simulink library "lib_all"  (Simulink 7.6)
settings: Solver=ode45  SolverMode=SingleTasking  StartTime=0.0  StopTime=10.0  RelTol=1e-3
values from neighbouring files (symbols this model uses):
  map_lib_03 = [6.8679 7.0159 7.4571 8.1849 9.1892 10.456 11.97 13.71 15.655 17.781 20.063 22.474 ... (803 elements, 73x11)]    [scr_simdata.mat]    (also in scr_simdata_all.mat)
  map_lib_06 = [2.5784 2.6727 2.9588 3.4453 4.1444 5.068 6.226 7.6242 9.2634 11.139 13.24 15.552 ... (803 elements, 73x11)]    [scr_simdata.mat]    (also in scr_simdata_all.mat)
  map_lib_09 = [0.0025784 0.0026727 0.0029588 0.0034453 0.0041444 0.005068 0.006226 0.0076242 0.0092634 0.011139 0.01324 0.015552 ... (803 elements, 73x11)]    [scr_simdata.mat]    (also in scr_simdata_all.mat)
  map_lib_10 = [2.74 2.75 2.76 2.77 2.78 2.79 2.8 2.81 2.82 2.83 2.84 2.85 ... (81 elements, 1x81)]    [scr_simdata.mat]    (also in scr_simdata_all.mat)
  map_lib_11 = [1.1983 1.2027 1.207 1.2113 1.2156 1.2198 1.2241 1.2283 1.2326 1.2368 1.241 1.2451 ... (81 elements, 1x81)]    [scr_simdata.mat]    (also in scr_simdata_all.mat)
  map_lib_12 = [2.74 2.75 2.76 2.77 2.78 2.79 2.8 2.81 2.82 2.83 2.84 2.85 ... (81 elements, 1x81)]    [scr_simdata.mat]    (also in scr_simdata_all.mat)
  map_lib_13 = [1.74 1.75 1.76 1.77 1.78 1.79 1.8 1.81 1.82 1.83 1.84 1.85 ... (81 elements, 1x81)]    [scr_simdata.mat]    (also in scr_simdata_all.mat)
  map_lib_16 = [0.00048828125 1.6159 1.8962 2.0821 2.2251 2.3426 2.4433 2.5318 2.611 2.683 2.749 2.8101 ... (1419 elements, 129x11)]    [scr_simdata.mat]    (also in scr_simdata_all.mat)
  map_mr2_01 = [0 800 1600 2400 3200 4000]    [scr_simdata.mat]    (also in scr_simdata_all.mat)
  map_mr2_02 = [1.32 1.32 1.33 1.34 1.36 1.38]    [scr_simdata.mat]    (also in scr_simdata_all.mat)
  map_mr2_03 = [0 800 1600 2400 3200 4000]    [scr_simdata.mat]    (also in scr_simdata_all.mat)
  map_mr2_04 = [1.32 1.32 1.33 1.34 1.36 1.38]    [scr_simdata.mat]    (also in scr_simdata_all.mat)
  map_mr2_05 = [0 800 1600 2400 3200 4000]    [scr_simdata.mat]    (also in scr_simdata_all.mat)
  map_mr2_06 = [1.32 1.32 1.33 1.34 1.36 1.38]    [scr_simdata.mat]    (also in scr_simdata_all.mat)
  Parameter1 = 6    [scr_simdata.mat]    (also in scr_simdata_all.mat)
  Parameter101 = 30    [scr_simdata.mat]    (also in scr_simdata_all.mat)
  Parameter102 = 12    [scr_simdata.mat]    (also in scr_simdata_all.mat)
  Parameter103 = -240    [scr_simdata.mat]    (also in scr_simdata_all.mat)
  Parameter108 = 48    [scr_simdata.mat]    (also in scr_simdata_all.mat)
  Parameter120 = 16    [scr_simdata.mat]    (also in scr_simdata_all.mat)
  Parameter121 = 87    [scr_simdata.mat]    (also in scr_simdata_all.mat)
  Parameter123 = 78    [scr_simdata.mat]    (also in scr_simdata_all.mat)
  Parameter124 = 60    [scr_simdata.mat]    (also in scr_simdata_all.mat)
  Parameter125 = -480    [scr_simdata.mat]    (also in scr_simdata_all.mat)
  Parameter130 = 96    [scr_simdata.mat]    (also in scr_simdata_all.mat)
  Parameter131 = 3    [scr_simdata.mat]    (also in scr_simdata_all.mat)
  Parameter144 = 3    [scr_simdata.mat]    (also in scr_simdata_all.mat)
  Parameter18 = 128    [scr_simdata.mat]    (also in scr_simdata_all.mat)
  Parameter19 = 16    [scr_simdata.mat]    (also in scr_simdata_all.mat)
  Parameter2 = 12    [scr_simdata.mat]    (also in scr_simdata_all.mat)
  Parameter20 = 7    [scr_simdata.mat]    (also in scr_simdata_all.mat)
  Parameter21 = 4    [scr_simdata.mat]    (also in scr_simdata_all.mat)
  Parameter3 = 18    [scr_simdata.mat]    (also in scr_simdata_all.mat)
  Parameter32 = 1    [scr_simdata.mat]    (also in scr_simdata_all.mat)
  Parameter34 = 24    [scr_simdata.mat]    (also in scr_simdata_all.mat)
  Parameter4 = 138    [scr_simdata.mat]    (also in scr_simdata_all.mat)
  Parameter42 = 48    [scr_simdata.mat]    (also in scr_simdata_all.mat)
  Parameter5 = 135    [scr_simdata.mat]    (also in scr_simdata_all.mat)
  Parameter55 = 0    [scr_simdata.mat]    (also in scr_simdata_all.mat)
  Parameter56 = 0    [scr_simdata.mat]    (also in scr_simdata_all.mat)
  Parameter57 = 1    [scr_simdata.mat]    (also in scr_simdata_all.mat)
  Parameter58 = 1    [scr_simdata.mat]    (also in scr_simdata_all.mat)
  Parameter59 = 2    [scr_simdata.mat]    (also in scr_simdata_all.mat)
  Parameter6 = 132    [scr_simdata.mat]    (also in scr_simdata_all.mat)
  Parameter60 = 2    [scr_simdata.mat]    (also in scr_simdata_all.mat)
  Parameter63 = 144    [scr_simdata.mat]    (also in scr_simdata_all.mat)
  Parameter7 = 126    [scr_simdata.mat]    (also in scr_simdata_all.mat)
  Parameter76 = 64    [scr_simdata.mat]    (also in scr_simdata_all.mat)
  Parameter77 = 135    [scr_simdata.mat]    (also in scr_simdata_all.mat)
  Parameter79 = 126    [scr_simdata.mat]    (also in scr_simdata_all.mat)
  Parameter80 = 108    [scr_simdata.mat]    (also in scr_simdata_all.mat)
  Parameter81 = 0    [scr_simdata.mat]    (also in scr_simdata_all.mat)
  Parameter86 = 0    [scr_simdata.mat]    (also in scr_simdata_all.mat)
  Parameter98 = 112    [scr_simdata.mat]    (also in scr_simdata_all.mat)
  Parameter99 = 39    [scr_simdata.mat]    (also in scr_simdata_all.mat)
undefined symbols (not defined in the file or in its folder): cylvolkp_hih_map2h, cylvolkp_map2h, pk1k_map2h
libraries: simulink x2
(part 1 of 36)
settings Model: autoc_cdm_savetype=ParentModel  autoc_cdm_name=database_dummy  autoc_current_model=lib_all  Creator=112454  LastModifiedBy=fukudak

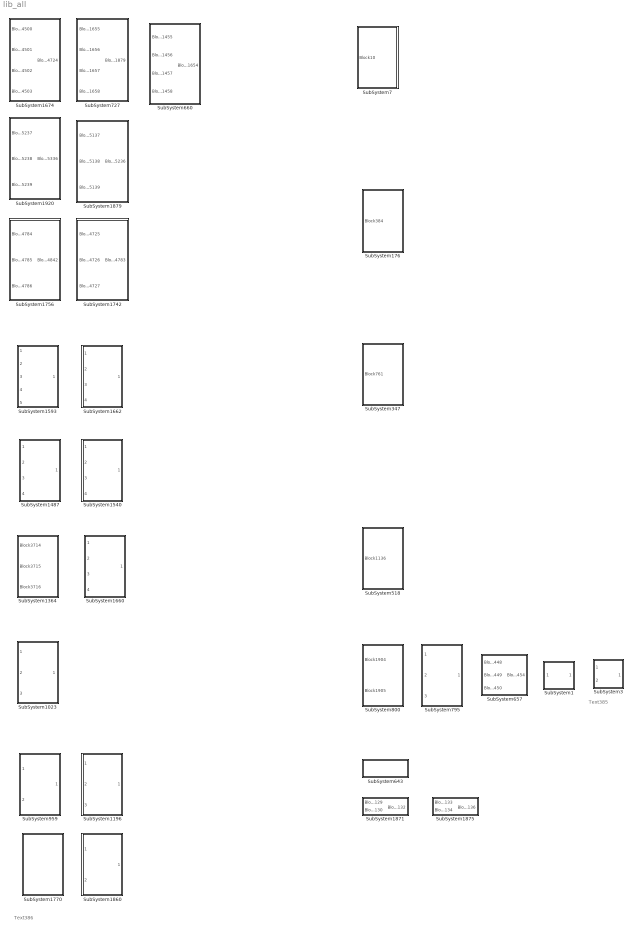
== system lib_all ==
  note: Text386
  note: Text385
  SubSystem1 : Subsystem  TreatAsAtomicUnit=on
  SubSystem1023 : Subsystem  TreatAsAtomicUnit=on
      settings: AttributesFormatString=Attribute523  RTWFcnNameOpts=Use subsystem name
  SubSystem1196 : Subsystem  TreatAsAtomicUnit=on
      settings: AttributesFormatString=Attribute636  RTWFcnNameOpts=Use subsystem name
  SubSystem1364 : Subsystem  TreatAsAtomicUnit=on
      settings: AttributesFormatString=Attribute739  RTWFcnNameOpts=Use subsystem name
  SubSystem1487 : Subsystem  TreatAsAtomicUnit=on
      settings: AttributesFormatString=Attribute793  RTWFcnNameOpts=Use subsystem name
  SubSystem1540 : Subsystem  TreatAsAtomicUnit=on
      settings: AttributesFormatString=Attribute812  RTWFcnNameOpts=Use subsystem name
  SubSystem1593 : Subsystem  TreatAsAtomicUnit=on
      settings: AttributesFormatString=Attribute831  RTWFcnNameOpts=Use subsystem name
  SubSystem1660 : Subsystem  TreatAsAtomicUnit=on
      settings: AttributesFormatString=Attribute864  RTWFcnNameOpts=Use subsystem name
  SubSystem1662 : Subsystem  TreatAsAtomicUnit=on
      settings: AttributesFormatString=Attribute867  RTWFcnNameOpts=Use subsystem name
  SubSystem1674 : Subsystem  TreatAsAtomicUnit=on
      settings: AttributesFormatString=Attribute880  RTWFcnNameOpts=Use subsystem name
  SubSystem1742 : Subsystem  TreatAsAtomicUnit=on
      settings: AttributesFormatString=Attribute917  RTWFcnNameOpts=Use subsystem name
  SubSystem1756 : Subsystem  TreatAsAtomicUnit=on
      settings: AttributesFormatString=Attribute931  RTWFcnNameOpts=Use subsystem name
  SubSystem176 : Subsystem  TreatAsAtomicUnit=on
      settings: AttributesFormatString=Attribute82  RTWFcnNameOpts=Use subsystem name
  SubSystem1770 : Subsystem  TreatAsAtomicUnit=on
      settings: AttributesFormatString=Attribute945  RTWFcnNameOpts=Use subsystem name
  SubSystem1860 : Subsystem  TreatAsAtomicUnit=on
      settings: AttributesFormatString=Attribute1028  RTWFcnNameOpts=Use subsystem name
  SubSystem1871 : Subsystem
  SubSystem1875 : Subsystem
  SubSystem1879 : Subsystem  TreatAsAtomicUnit=on
      settings: AttributesFormatString=Attribute1038  RTWFcnNameOpts=Use subsystem name
  SubSystem1920 : Subsystem  TreatAsAtomicUnit=on
      settings: AttributesFormatString=Attribute1054  RTWFcnNameOpts=Use subsystem name
  SubSystem3 : Subsystem
  SubSystem347 : Subsystem  TreatAsAtomicUnit=on
      settings: AttributesFormatString=Attribute164  RTWFcnNameOpts=Use subsystem name
  SubSystem518 : Subsystem  TreatAsAtomicUnit=on
      settings: AttributesFormatString=Attribute245  RTWFcnNameOpts=Use subsystem name
  SubSystem643 : Subsystem  TreatAsAtomicUnit=on  RTWFileName=gacrnk_get
      settings: RTWFcnNameOpts=Use subsystem name
  SubSystem657 : Subsystem  TreatAsAtomicUnit=on
      settings: RTWSystemCode=Inline  RTWFcnNameOpts=Use subsystem name
  SubSystem660 : Subsystem  TreatAsAtomicUnit=on
      settings: AttributesFormatString=Attribute306  RTWFcnNameOpts=Use subsystem name
  SubSystem7 : Subsystem  TreatAsAtomicUnit=on
      settings: AttributesFormatString=Attribute2  RTWFcnNameOpts=Use subsystem name
  SubSystem727 : Subsystem  TreatAsAtomicUnit=on
      settings: AttributesFormatString=Attribute342  RTWFcnNameOpts=Use subsystem name
  SubSystem795 : Subsystem  TreatAsAtomicUnit=on
      settings: AttributesFormatString=Attribute379  RTWFcnNameOpts=Use subsystem name
  SubSystem800 : Subsystem  TreatAsAtomicUnit=on
      settings: AttributesFormatString=Attribute386  RTWFcnNameOpts=Use subsystem name
  SubSystem959 : Subsystem  TreatAsAtomicUnit=on
      settings: AttributesFormatString=Attribute491  RTWFcnNameOpts=Use subsystem name

== system lib_all/SubSystem1 ==
  note: Text1
  Block1 : Inport
  Block2 : Constant  Value=Parameter63  OutDataTypeStr=double  SampleTime=-1
  Block3 : Sum  Inputs=+-  OutDataTypeStr=double  RndMeth=Zero  SaturateOnIntegerOverflow=on  InputSameDT=on
  Block4 : Switch  Threshold=0.5  RndMeth=Zero  SaturateOnIntegerOverflow=on  InputSameDT=on
  SubSystem2 : Subsystem
  Block5 : Outport
  wires:
  Block2/1 -> Block3/2, SubSystem2/2(In2)
  SubSystem2/1(Out1) -> Block4/2
  Block3/1 -> Block4/1
  Block1/1 -> SubSystem2/1(In1), Block3/1, Block4/3
  Block4/1 -> Block5/1

== system lib_all/SubSystem1023 ==
  note: Text236
  Block2623 : Inport
  Block2624 : Inport  Port=2
  Block2625 : Inport  Port=3
  Block2626 : Constant  Value=0  OutDataTypeStr=double  SampleTime=-1
  Block2627 : Constant  Value=0  OutDataTypeStr=double  SampleTime=-1
  Block2628 : Sum  Inputs=-+  OutDataTypeStr=double  RndMeth=Zero  SaturateOnIntegerOverflow=on  InputSameDT=on
  Block2629 : Sum  Inputs=+-  OutDataTypeStr=double  RndMeth=Zero  SaturateOnIntegerOverflow=on  InputSameDT=on
  SubSystem1024 : Subsystem  TreatAsAtomicUnit=on  mask(***ATC.DIVISION***){bd_version=1.01.A, r0=チェック無し, r1=, r2=, r3=, r4=, r5=, r6=, r7=, r8=, r9=, c0=, c1=, c2=, c3=, c4=, c5=, c6=, c7=, c8=, c9=}
      mask prompts: bd_version "Version"
      mask help: これはAutoCからのブロックです．編集禁止．
      settings: OpenFcn=warndlg('変更禁止!');
      mask Help: web(ui_blockhelpmng(gcb));
  SubSystem1025 : Subsystem  Priority=1  TreatAsAtomicUnit=on
      settings: AttributesFormatString=Attribute526  RTWFcnNameOpts=Use subsystem name
  SubSystem1093 : Subsystem  Priority=2  TreatAsAtomicUnit=on
      settings: AttributesFormatString=Attribute563  RTWFcnNameOpts=Use subsystem name
  SubSystem1161 : Subsystem  Priority=3  TreatAsAtomicUnit=on
      settings: AttributesFormatString=Attribute600  RTWFcnNameOpts=Use subsystem name
  SubSystem1175 : Subsystem  Priority=4  TreatAsAtomicUnit=on
      settings: AttributesFormatString=Attribute614  RTWFcnNameOpts=Use subsystem name
  SubSystem1189 : Subsystem
  Block3216 : Outport
  wires:
  Block2626/1 -> SubSystem1025/4(Block2633)
  Block2627/1 -> SubSystem1093/4(Block2858)
  SubSystem1189/1(Block3215) -> SubSystem1025/3(Block2632), SubSystem1093/3(Block2857), SubSystem1175/3(Block3141), SubSystem1161/3(Block3082)
  Block2625/1 -> SubSystem1175/2(Block3140), SubSystem1093/2(Block2856)
  Block2624/1 -> SubSystem1161/2(Block3081), SubSystem1025/2(Block2631)
  SubSystem1024/1(Out) -> Block3216/1
  Block2629/1 -> SubSystem1024/2(div)
  Block2628/1 -> SubSystem1024/1(mult)
  SubSystem1175/1(Block3197) -> Block2629/2
  SubSystem1161/1(Block3138) -> Block2629/1
  SubSystem1093/1(Block3079) -> Block2628/2
  SubSystem1025/1(Block2854) -> Block2628/1
  Block2623/1 -> SubSystem1025/1(Block2630), SubSystem1093/1(Block2855), SubSystem1175/1(Block3139), SubSystem1161/1(Block3080), SubSystem1189/1(Block3198)

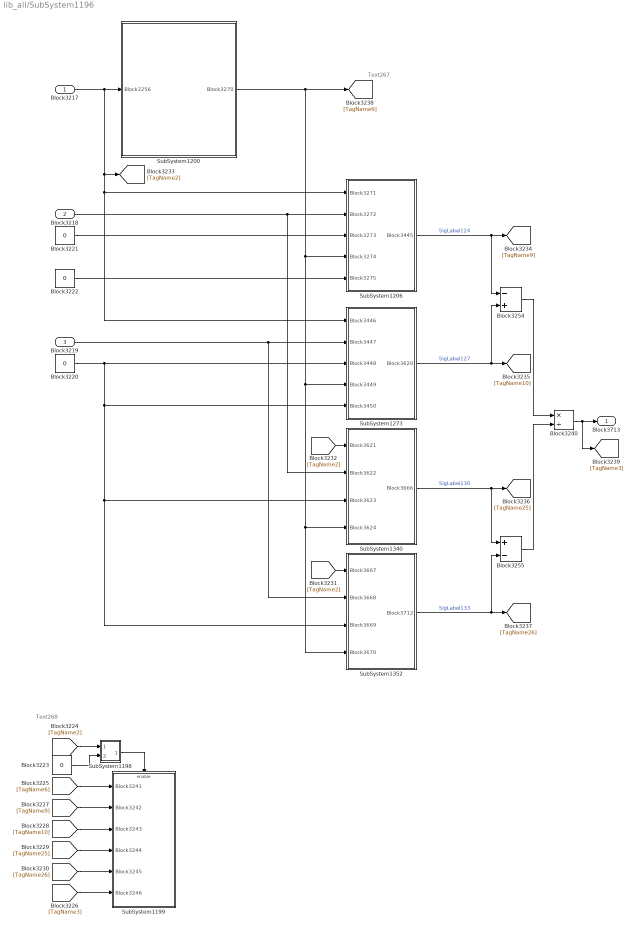
== system lib_all/SubSystem1196 ==
  note: Text268
  note: Text267
  Block3217 : Inport
  Block3218 : Inport  Port=2
  Block3219 : Inport  Port=3
  Block{3220..3223} (4 alike) : Constant  Value=0  OutDataTypeStr=double  SampleTime=-1
  Block3240 : Product  Inputs=*/  OutDataTypeStr=double  SaturateOnIntegerOverflow=on  RndMeth=Zero  InputSameDT=on
  Block3254 : Sum  Inputs=-+  OutDataTypeStr=double  RndMeth=Zero  SaturateOnIntegerOverflow=on  InputSameDT=on
  Block3255 : Sum  Inputs=+-  OutDataTypeStr=double  RndMeth=Zero  SaturateOnIntegerOverflow=on  InputSameDT=on
  SubSystem1198 : Subsystem
  SubSystem1199 : Subsystem
  SubSystem1200 : Subsystem
  SubSystem1206 : Subsystem  Priority=1  TreatAsAtomicUnit=on
      settings: AttributesFormatString=Attribute647  RTWFcnNameOpts=Use subsystem name
  SubSystem1273 : Subsystem  Priority=2  TreatAsAtomicUnit=on
      settings: AttributesFormatString=Attribute680  RTWFcnNameOpts=Use subsystem name
  SubSystem1340 : Subsystem  Priority=3  TreatAsAtomicUnit=on
      settings: AttributesFormatString=Attribute713  RTWFcnNameOpts=Use subsystem name
  SubSystem1352 : Subsystem  Priority=4  TreatAsAtomicUnit=on
      settings: AttributesFormatString=Attribute726  RTWFcnNameOpts=Use subsystem name
  Block3713 : Outport
  wires:
  [TagName2] -> SubSystem1340/1(Block3621)
  [TagName2] -> SubSystem1352/1(Block3667)
  SubSystem1198/1(Out1) -> SubSystem1199/Enable
  [TagName26] -> SubSystem1199/5(Block3245)
  [TagName25] -> SubSystem1199/4(Block3244)
  [TagName10] -> SubSystem1199/3(Block3243)
  [TagName9] -> SubSystem1199/2(Block3242)
  [TagName3] -> SubSystem1199/6(Block3246)
  [TagName6] -> SubSystem1199/1(Block3241)
  Block3223/1 -> SubSystem1198/2(In2)
  [TagName2] -> SubSystem1198/1(In1)
  Block3240/1 -> Block3713/1, [TagName3]
  Block3255/1 -> Block3240/2
  Block3254/1 -> Block3240/1
  SubSystem1352/1(Block3712) -> Block3255/2, [TagName26]  "SigLabel133"
  SubSystem1340/1(Block3666) -> Block3255/1, [TagName25]  "SigLabel130"
  SubSystem1273/1(Block3620) -> Block3254/2, [TagName10]  "SigLabel127"
  SubSystem1206/1(Block3445) -> Block3254/1, [TagName9]  "SigLabel124"
  Block3220/1 -> SubSystem1273/3(Block3448), SubSystem1273/5(Block3450), SubSystem1352/3(Block3669), SubSystem1340/3(Block3623)
  Block3219/1 -> SubSystem1352/2(Block3668), SubSystem1273/2(Block3447)
  SubSystem1200/1(Block3270) -> SubSystem1206/4(Block3274), SubSystem1352/4(Block3670), SubSystem1340/4(Block3624), SubSystem1273/4(Block3449), [TagName6]
  Block3222/1 -> SubSystem1206/5(Block3275)
  Block3221/1 -> SubSystem1206/3(Block3273)
  Block3218/1 -> SubSystem1340/2(Block3622), SubSystem1206/2(Block3272)
  Block3217/1 -> SubSystem1200/1(Block3256), SubSystem1273/1(Block3446), SubSystem1206/1(Block3271), [TagName2]

== system lib_all/SubSystem1364 ==
  note: Text289
  note: Text288
  note: Text287
  note: Text286
  Block3714 : Inport
  Block3715 : Inport  Port=2
  Block3716 : Inport  Port=3
  Block3717 : Constant  Value=0  OutDataTypeStr=double  SampleTime=-1
  Block3718 : Constant  OutDataTypeStr=double  SampleTime=-1
  Block3719 : Constant  Value=0  OutDataTypeStr=double  SampleTime=-1
  Block3720 : DataStoreRead  DataStoreName=DSM15
  Block3721 : DataStoreWrite  DataStoreName=DSM101
  Block3730 : Sum  OutDataTypeStr=double  RndMeth=Zero  SaturateOnIntegerOverflow=on  InputSameDT=on
  SubSystem1366 : Subsystem  Priority=1  TreatAsAtomicUnit=on
      settings: AttributesFormatString=Attribute743  RTWFcnNameOpts=Use subsystem name
  SubSystem1419 : Subsystem  Priority=2  TreatAsAtomicUnit=on
      settings: AttributesFormatString=Attribute762  RTWFcnNameOpts=Use subsystem name
  SubSystem1472 : Subsystem
  SubSystem1478 : Subsystem  TreatAsAtomicUnit=on
      settings: RTWFcnNameOpts=Use subsystem name
  SubSystem1480 : Subsystem  TreatAsAtomicUnit=on  mask(***ATC.DSWVEC***){bd_version=1.01.A, r0=DSM99, r1=-1, r2=, r3=, r4=, r5=, r6=, r7=, r8=, r9=, c0=, c1=, c2=, c3=, c4=, c5=, c6=, c7=, c8=, c9=}
      mask prompts: bd_version "Version"
      mask help: これはAutoCからのブロックです．編集禁止．
      settings: OpenFcn=warndlg('変更禁止!');
      mask Help: web(ui_blockhelpmng(gcb));
      mask Display: disp('ATC.DSRVEC')
  SubSystem1481 : Subsystem  TreatAsAtomicUnit=on  mask(***ATC.DSWVEC***){bd_version=1.01.A, r0=DSM100, r1=-1, r2=, r3=, r4=, r5=, r6=, r7=, r8=, r9=, c0=, c1=, c2=, c3=, c4=, c5=, c6=, c7=, c8=, c9=}
      mask prompts: bd_version "Version"
      mask help: これはAutoCからのブロックです．編集禁止．
      settings: OpenFcn=warndlg('変更禁止!');
      mask Help: web(ui_blockhelpmng(gcb));
      mask Display: disp('ATC.DSRVEC')
  SubSystem1482 : Subsystem  TreatAsAtomicUnit=on  mask(***ATC.DSRVEC***){bd_version=1.01.A, r0=DSM98, r1=-1, r2=, r3=, r4=, r5=, r6=, r7=, r8=, r9=, c0=, c1=, c2=, c3=, c4=, c5=, c6=, c7=, c8=, c9=}
      mask prompts: bd_version "Version"
      mask help: これはAutoCからのブロックです．編集禁止．
      settings: OpenFcn=warndlg('変更禁止!');
      mask Help: web(ui_blockhelpmng(gcb));
      mask Display: disp('ATC.DSRVEC')
  SubSystem1483 : Subsystem  TreatAsAtomicUnit=on  mask(***ATC.DSWVEC***){bd_version=1.01.A, r0=DSM41, r1=-1, r2=, r3=, r4=, r5=, r6=, r7=, r8=, r9=, c0=, c1=, c2=, c3=, c4=, c5=, c6=, c7=, c8=, c9=}
      mask prompts: bd_version "Version"
      mask help: これはAutoCからのブロックです．編集禁止．
      settings: OpenFcn=warndlg('変更禁止!');
      mask Help: web(ui_blockhelpmng(gcb));
      mask Display: disp('ATC.DSRVEC')
  SubSystem1484 : Subsystem  TreatAsAtomicUnit=on  mask(***ATC.DSRVEC***){bd_version=1.01.A, r0=DSM42, r1=-1, r2=, r3=, r4=, r5=, r6=, r7=, r8=, r9=, c0=, c1=, c2=, c3=, c4=, c5=, c6=, c7=, c8=, c9=}
      mask prompts: bd_version "Version"
      mask help: これはAutoCからのブロックです．編集禁止．
      settings: OpenFcn=warndlg('変更禁止!');
      mask Help: web(ui_blockhelpmng(gcb));
      mask Display: disp('ATC.DSRVEC')
  SubSystem1485 : Subsystem  TreatAsAtomicUnit=on  mask(***ATC.DSWVEC***){bd_version=1.01.A, r0=DSM42, r1=-1, r2=, r3=, r4=, r5=, r6=, r7=, r8=, r9=, c0=, c1=, c2=, c3=, c4=, c5=, c6=, c7=, c8=, c9=}
      mask prompts: bd_version "Version"
      mask help: これはAutoCからのブロックです．編集禁止．
      settings: OpenFcn=warndlg('変更禁止!');
      mask Help: web(ui_blockhelpmng(gcb));
      mask Display: disp('ATC.DSRVEC')
  SubSystem1486 : Subsystem  TreatAsAtomicUnit=on
  wires:
  [TagName17] -> SubSystem1480/1(input)
  [TagName16] -> SubSystem1481/1(input)
  [TagName5] -> SubSystem1480/2(element), SubSystem1481/2(element)
  [TagName15] -> Block3721/1
  Block3730/1 -> SubSystem1472/1(Block3975), SubSystem1485/2(element), SubSystem1483/2(element), SubSystem1482/1(element), SubSystem1484/1(element), [TagName5]
  SubSystem1472/1(Block3994) -> SubSystem1478/4(Block3999), [TagName15]
  SubSystem1486/1(Block4019) -> SubSystem1485/1(input)
  Block3720/1 -> SubSystem1486/3(Block4013)
  SubSystem1484/1(Output) -> SubSystem1486/1(Block4011)
  SubSystem1478/1(Block4010) -> SubSystem1486/2(Block4012), SubSystem1483/1(input)
  SubSystem1482/1(Output) -> SubSystem1478/3(Block3998)
  SubSystem1419/1(Block3974) -> SubSystem1478/2(Block3997), [TagName17]
  SubSystem1366/1(Block3852) -> SubSystem1478/1(Block3996), [TagName16]
  SubSystem1472/2(Block3995) -> SubSystem1419/4(Block3856), SubSystem1366/4(Block3734)
  Block3717/1 -> SubSystem1366/3(Block3733)
  Block3719/1 -> SubSystem1419/3(Block3855)
  Block3716/1 -> SubSystem1419/2(Block3854)
  Block3715/1 -> SubSystem1366/2(Block3732)
  Block3718/1 -> Block3730/2
  Block3714/1 -> Block3730/1, SubSystem1419/1(Block3853), SubSystem1366/1(Block3731)

== system lib_all/SubSystem1487 ==
  note: Text295
  Block4020 : Inport
  Block4021 : Inport  Port=2
  Block4022 : Inport  Port=3
  Block4023 : Inport  Port=4
  Block4024 : Constant  Value=0  OutDataTypeStr=double  SampleTime=-1
  Block4025 : Constant  OutDataTypeStr=double  SampleTime=-1
  Block4026 : Constant  Value=0  OutDataTypeStr=double  SampleTime=-1
  Block4027 : Gain  Gain=1/5  SaturateOnIntegerOverflow=on  OutDataTypeStr=Inherit: Same as input  ParamDataTypeStr=Inherit: Same as input
      settings: AttributesFormatString=Attribute797
  Block4028 : Product  OutDataTypeStr=double  SaturateOnIntegerOverflow=on  RndMeth=Zero  InputSameDT=on
  Block4029 : Sum  Inputs=-+  OutDataTypeStr=double  RndMeth=Zero  SaturateOnIntegerOverflow=on  InputSameDT=on
  Block4030 : Sum  OutDataTypeStr=double  RndMeth=Zero  SaturateOnIntegerOverflow=on  InputSameDT=on
  Block4031 : Sum  OutDataTypeStr=double  RndMeth=Zero  SaturateOnIntegerOverflow=on  InputSameDT=on
  Block4032 : Switch  Threshold=0.5  RndMeth=Zero  SaturateOnIntegerOverflow=on  InputSameDT=on
  SubSystem1489 : Subsystem
  SubSystem1490 : Subsystem
  SubSystem1496 : Subsystem
  Block4141 : Outport
  wires:
  Block4031/1 -> SubSystem1496/3(Block4062), SubSystem1490/2(Block4034)
  Block4020/1 -> Block4031/1
  Block4027/1 -> Block4030/2
  Block4028/1 -> Block4027/1
  Block4025/1 -> Block4031/2
  Block4024/1 -> Block4032/1
  Block4032/1 -> Block4141/1
  SubSystem1489/1(Out1) -> Block4032/2
  Block4026/1 -> SubSystem1489/2(In2)
  Block4022/1 -> SubSystem1490/3(Block4035)
  Block4030/1 -> SubSystem1489/1(In1), Block4032/3
  SubSystem1490/2(Block4059) -> Block4028/2
  Block4029/1 -> Block4028/1  "SigLabel142"
  SubSystem1496/2(Block4140) -> Block4029/2
  SubSystem1496/1(Block4139) -> Block4029/1, Block4030/1
  Block4023/1 -> SubSystem1496/2(Block4061)
  SubSystem1490/1(Block4058) -> SubSystem1496/1(Block4060)
  Block4021/1 -> SubSystem1490/1(Block4033)

== system lib_all/SubSystem1540 ==
  note: Text301
  Block4142 : Inport
  Block4143 : Inport  Port=2
  Block4144 : Inport  Port=3
  Block4145 : Inport  Port=4
  Block4146 : Constant  Value=0  OutDataTypeStr=double  SampleTime=-1
  Block4147 : Constant  OutDataTypeStr=double  SampleTime=-1
  Block4148 : Constant  Value=0  OutDataTypeStr=double  SampleTime=-1
  Block4149 : Gain  Gain=1/5  SaturateOnIntegerOverflow=on  OutDataTypeStr=Inherit: Same as input  ParamDataTypeStr=Inherit: Same as input
      settings: AttributesFormatString=Attribute816
  Block4150 : Product  OutDataTypeStr=double  SaturateOnIntegerOverflow=on  RndMeth=Zero  InputSameDT=on
  Block4151 : Sum  Inputs=-+  OutDataTypeStr=double  RndMeth=Zero  SaturateOnIntegerOverflow=on  InputSameDT=on
  Block4152 : Sum  OutDataTypeStr=double  RndMeth=Zero  SaturateOnIntegerOverflow=on  InputSameDT=on
  Block4153 : Sum  OutDataTypeStr=double  RndMeth=Zero  SaturateOnIntegerOverflow=on  InputSameDT=on
  Block4154 : Switch  Threshold=0.5  RndMeth=Zero  SaturateOnIntegerOverflow=on  InputSameDT=on
  SubSystem1542 : Subsystem
  SubSystem1543 : Subsystem
  SubSystem1549 : Subsystem
  Block4263 : Outport
  wires:
  Block4143/1 -> SubSystem1543/1(Block4155)
  SubSystem1543/1(Block4180) -> SubSystem1549/1(Block4182)
  Block4145/1 -> SubSystem1549/2(Block4183)
  SubSystem1549/1(Block4261) -> Block4152/1, Block4151/1
  SubSystem1549/2(Block4262) -> Block4151/2
  Block4151/1 -> Block4150/1  "SigLabel145"
  SubSystem1543/2(Block4181) -> Block4150/2
  Block4152/1 -> Block4154/3, SubSystem1542/1(In1)
  Block4144/1 -> SubSystem1543/3(Block4157)
  Block4148/1 -> SubSystem1542/2(In2)
  SubSystem1542/1(Out1) -> Block4154/2
  Block4154/1 -> Block4263/1
  Block4146/1 -> Block4154/1
  Block4147/1 -> Block4153/2
  Block4150/1 -> Block4149/1
  Block4149/1 -> Block4152/2
  Block4142/1 -> Block4153/1
  Block4153/1 -> SubSystem1543/2(Block4156), SubSystem1549/3(Block4184)

== system lib_all/SubSystem1593 ==
  note: Text313
  note: Text312
  note: Text311
  Block4264 : Inport
  Block4265 : Inport  Port=2
  Block4266 : Inport  Port=3
  Block4267 : Inport  Port=4
  Block4268 : Inport  Port=5
  Block4269 : Product  OutDataTypeStr=double  SaturateOnIntegerOverflow=on  RndMeth=Zero  InputSameDT=on
  SubSystem1595 : Subsystem  Priority=1  TreatAsAtomicUnit=on
      settings: AttributesFormatString=Attribute832  RTWFcnNameOpts=Use subsystem name
  SubSystem1648 : Subsystem  Priority=2  TreatAsAtomicUnit=on
      settings: AttributesFormatString=Attribute851  RTWFcnNameOpts=Use subsystem name
  Block4438 : Outport
  wires:
  Block4267/1 -> SubSystem1648/4(Block4395)
  Block4268/1 -> SubSystem1595/4(Block4273)
  Block4266/1 -> SubSystem1595/3(Block4272), SubSystem1648/3(Block4394)
  Block4269/1 -> Block4438/1
  SubSystem1648/1(Block4437) -> Block4269/2  "SigLabel151"
  SubSystem1595/1(Block4391) -> Block4269/1  "SigLabel150"
  Block4265/1 -> SubSystem1595/2(Block4271), SubSystem1648/2(Block4393)
  Block4264/1 -> SubSystem1595/1(Block4270), SubSystem1648/1(Block4392)

== system lib_all/SubSystem1660 ==
  note: Text316
  note: Text315
  note: Text314
  Block4439 : Inport
  Block4440 : Inport  Port=2
  Block4441 : Inport  Port=3
  Block4442 : Inport  Port=4
  Block4443 : DataStoreWrite  DataStoreName=DSM90
  Block4444 : DataStoreWrite  DataStoreName=DSM91
  Block4449 : Product  OutDataTypeStr=double  SaturateOnIntegerOverflow=on  RndMeth=Zero  InputSameDT=on
  Block4450 : Product  Inputs=*/  OutDataTypeStr=double  SaturateOnIntegerOverflow=on  RndMeth=Zero  InputSameDT=on
  Block4451 : Lookup2D  RowIndex=pk1k_map2h_y  ColumnIndex=pk1k_map2h_x  Table=pk1k_map2h  SaturateOnIntegerOverflow=on  InputSameDT=on
      settings: AttributesFormatString=<%<OutputValues>>\nAttribute865
  Block4452 : Lookup2D  RowIndex=pk1k_map2h_y  ColumnIndex=pk1k_map2h_x  Table=pk1k_map2h  SaturateOnIntegerOverflow=on  InputSameDT=on
      settings: AttributesFormatString=<%<OutputValues>>\nAttribute866
  Block4453 : Outport
  wires:
  Block4442/1 -> Block4451/2, Block4452/2
  [TagName20] -> Block4444/1
  [TagName19] -> Block4443/1
  Block4451/1 -> Block4450/2, [TagName19]
  Block4450/1 -> Block4453/1
  Block4449/1 -> Block4450/1
  Block4441/1 -> Block4449/2
  Block4452/1 -> Block4449/1, [TagName20]
  Block4439/1 -> Block4451/1
  Block4440/1 -> Block4452/1

== system lib_all/SubSystem1662 ==
  note: Text319
  Block4454 : Inport
  Block4455 : Inport  Port=2
  Block4456 : Inport  Port=3
  Block4457 : Inport  Port=4
  SubSystem1664 : Subsystem
  SubSystem1672 : Subsystem
  Block4499 : Outport
  wires:
  Block4456/1 -> SubSystem1664/3(Block4460)
  SubSystem1672/1(Block4498) -> Block4499/1
  Block4457/1 -> SubSystem1672/1(Block4491)
  SubSystem1664/1(Block4490) -> SubSystem1672/2(Block4492)
  Block4454/1 -> SubSystem1664/2(Block4459)
  Block4455/1 -> SubSystem1664/1(Block4458)

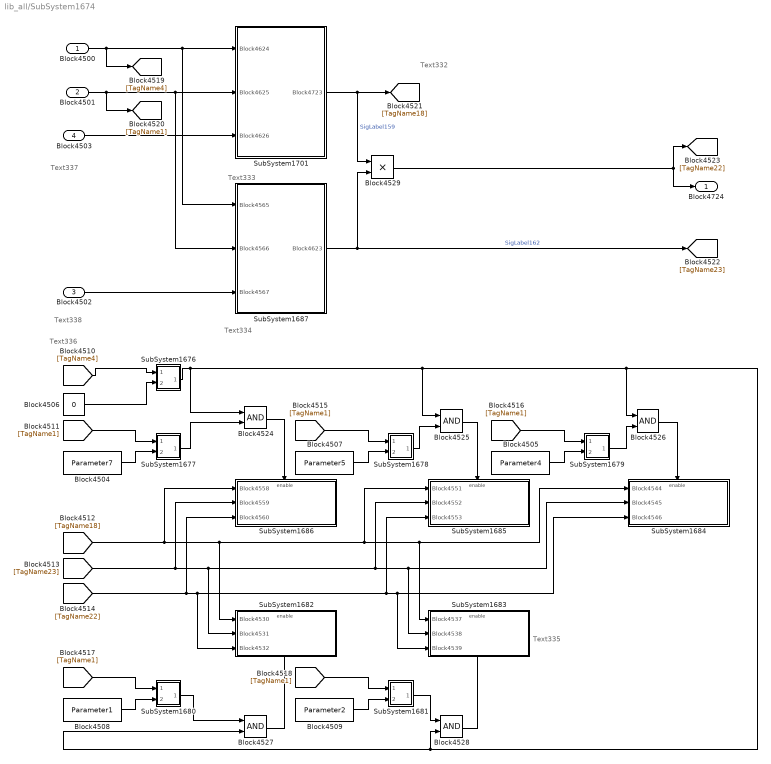
== system lib_all/SubSystem1674 ==
  note: Text338
  note: Text337
  note: Text336
  note: Text335
  note: Text334
  note: Text333
  note: Text332
  Block4500 : Inport
  Block4501 : Inport  Port=2
  Block4502 : Inport  Port=3
  Block4503 : Inport  Port=4
  Block4504 : Constant  Value=Parameter7  OutDataTypeStr=double  SampleTime=-1
  Block4505 : Constant  Value=Parameter4  OutDataTypeStr=double  SampleTime=-1
  Block4506 : Constant  Value=0  OutDataTypeStr=double  SampleTime=-1
  Block4507 : Constant  Value=Parameter5  OutDataTypeStr=double  SampleTime=-1
  Block4508 : Constant  Value=Parameter1  OutDataTypeStr=double  SampleTime=-1
  Block4509 : Constant  Value=Parameter2  OutDataTypeStr=double  SampleTime=-1
  Block{4524..4528} (5 alike) : Logic
  Block4529 : Product  OutDataTypeStr=double  SaturateOnIntegerOverflow=on  RndMeth=Zero  InputSameDT=on
  SubSystem1676 : Subsystem
  SubSystem1677 : Subsystem
  SubSystem1678 : Subsystem
  SubSystem1679 : Subsystem
  SubSystem1680 : Subsystem
  SubSystem1681 : Subsystem
  SubSystem1682 : Subsystem
      settings: PropExecContextOutsideSubsystem=on
  SubSystem1683 : Subsystem
      settings: PropExecContextOutsideSubsystem=on
  SubSystem1684 : Subsystem
      settings: PropExecContextOutsideSubsystem=on
  SubSystem1685 : Subsystem
      settings: PropExecContextOutsideSubsystem=on
  SubSystem1686 : Subsystem
      settings: PropExecContextOutsideSubsystem=on
  SubSystem1687 : Subsystem  Priority=2  TreatAsAtomicUnit=on
      settings: AttributesFormatString=Attribute887  RTWFcnNameOpts=Use subsystem name
  SubSystem1701 : Subsystem  Priority=1  TreatAsAtomicUnit=on
      settings: AttributesFormatString=Attribute901  RTWFcnNameOpts=Use subsystem name
  Block4724 : Outport
  wires:
  Block4503/1 -> SubSystem1701/3(Block4626)
  Block4502/1 -> SubSystem1687/3(Block4567)
  SubSystem1681/1(Out1) -> Block4528/1
  SubSystem1680/1(Out1) -> Block4527/1
  Block4528/1 -> SubSystem1683/Enable
  Block4527/1 -> SubSystem1682/Enable
  Block4509/1 -> SubSystem1681/2(In2)
  [TagName1] -> SubSystem1681/1(In1)
  Block4508/1 -> SubSystem1680/2(In2)
  [TagName1] -> SubSystem1680/1(In1)
  [TagName22] -> SubSystem1686/3(Block4560), SubSystem1682/3(Block4532), SubSystem1684/3(Block4546), SubSystem1683/3(Block4539), SubSystem1685/3(Block4553)
  [TagName23] -> SubSystem1686/2(Block4559), SubSystem1682/2(Block4531), SubSystem1684/2(Block4545), SubSystem1683/2(Block4538), SubSystem1685/2(Block4552)
  [TagName18] -> SubSystem1686/1(Block4558), SubSystem1682/1(Block4530), SubSystem1684/1(Block4544), SubSystem1683/1(Block4537), SubSystem1685/1(Block4551)
  Block4526/1 -> SubSystem1684/Enable
  Block4525/1 -> SubSystem1685/Enable
  SubSystem1679/1(Out1) -> Block4526/2
  Block4505/1 -> SubSystem1679/2(In2)
  [TagName1] -> SubSystem1679/1(In1)
  Block4524/1 -> SubSystem1686/Enable
  SubSystem1678/1(Out1) -> Block4525/2
  Block4507/1 -> SubSystem1678/2(In2)
  [TagName1] -> SubSystem1678/1(In1)
  SubSystem1677/1(Out1) -> Block4524/2
  SubSystem1676/1(Out1) -> Block4524/1, Block4525/1, Block4526/1, Block4528/2, Block4527/2
  Block4504/1 -> SubSystem1677/2(In2)
  [TagName1] -> SubSystem1677/1(In1)
  Block4506/1 -> SubSystem1676/2(In2)
  [TagName4] -> SubSystem1676/1(In1)
  Block4529/1 -> Block4724/1, [TagName22]
  SubSystem1687/1(Block4623) -> Block4529/2, [TagName23]  "SigLabel162"
  SubSystem1701/1(Block4723) -> Block4529/1, [TagName18]  "SigLabel159"
  Block4501/1 -> SubSystem1687/2(Block4566), SubSystem1701/2(Block4625), [TagName1]
  Block4500/1 -> SubSystem1687/1(Block4565), SubSystem1701/1(Block4624), [TagName4]

== system lib_all/SubSystem1742 ==
  note: Text342
  note: Text341
  Block4725 : Inport
  Block4726 : Inport  Port=2
  Block4727 : Inport  Port=3
  Block4728 : Constant  Value=0  OutDataTypeStr=double  SampleTime=-1
  SubSystem1744 : Subsystem
  SubSystem1745 : Subsystem
  SubSystem1753 : Subsystem  TreatAsAtomicUnit=on
  SubSystem1754 : Subsystem
  Block4783 : Outport
  wires:
  [TagName23] -> SubSystem1753/3(Block4770)
  [TagName11] -> SubSystem1753/2(Block4769)
  [TagName1] -> SubSystem1753/1(Block4768)
  SubSystem1744/1(Out1) -> SubSystem1753/Enable
  Block4728/1 -> SubSystem1744/2(In2)
  [TagName4] -> SubSystem1744/1(In1)
  SubSystem1754/1(Block4782) -> Block4783/1, [TagName23]
  Block4727/1 -> SubSystem1754/1(Block4775)
  SubSystem1745/1(Block4767) -> SubSystem1754/2(Block4776), [TagName11]
  Block4725/1 -> SubSystem1745/2(Block4738), [TagName4]
  Block4726/1 -> SubSystem1745/1(Block4737), [TagName1]

== system lib_all/SubSystem1756 ==
  note: Text346
  note: Text345
  Block4784 : Inport
  Block4785 : Inport  Port=2
  Block4786 : Inport  Port=3
  Block4787 : Constant  Value=0  OutDataTypeStr=double  SampleTime=-1
  SubSystem1758 : Subsystem
  SubSystem1759 : Subsystem
  SubSystem1767 : Subsystem  TreatAsAtomicUnit=on
  SubSystem1768 : Subsystem
  Block4842 : Outport
  wires:
  Block4785/1 -> [TagName1], SubSystem1759/1(Block4796)
  Block4784/1 -> [TagName4], SubSystem1759/2(Block4797)
  SubSystem1759/1(Block4826) -> [TagName11], SubSystem1768/2(Block4835)
  Block4786/1 -> SubSystem1768/1(Block4834)
  SubSystem1768/1(Block4841) -> [TagName23], Block4842/1
  [TagName4] -> SubSystem1758/1(In1)
  Block4787/1 -> SubSystem1758/2(In2)
  SubSystem1758/1(Out1) -> SubSystem1767/Enable
  [TagName1] -> SubSystem1767/1(Block4827)
  [TagName11] -> SubSystem1767/2(Block4828)
  [TagName23] -> SubSystem1767/3(Block4829)

== system lib_all/SubSystem176 ==
  note: Text52
  note: Text51
  note: Text50
  note: Text49
  Block384 : Inport
  SubSystem178 : Subsystem  Priority=1  TreatAsAtomicUnit=on
      settings: AttributesFormatString=Attribute83  RTWSystemCode=Inline
  SubSystem303 : Subsystem  Priority=3  TreatAsAtomicUnit=on
      settings: AttributesFormatString=Attribute134  RTWSystemCode=Inline
  SubSystem329 : Subsystem  Priority=4  TreatAsAtomicUnit=on
      settings: AttributesFormatString=Attribute151  RTWSystemCode=Inline
  SubSystem344 : Subsystem  Priority=2  TreatAsAtomicUnit=on
      settings: AttributesFormatString=Attribute161  RTWSystemCode=Inline
  wires:
  Block384/1 -> SubSystem178/1(Block385), SubSystem344/1(Block756), SubSystem303/1(Block685)

== system lib_all/SubSystem1770 ==
  note: Text365
  SubSystem1771 : Subsystem  TreatAsAtomicUnit=on
      settings: OpenFcn=warndlg('変更禁止!');
      mask Type: ***ATC.CMPLSW***
      mask Description: これはAutoCからのブロックです．編集禁止．
  SubSystem1772 : Subsystem  TreatAsAtomicUnit=on
      settings: OpenFcn=warndlg('変更禁止!');
      mask Type: ***ATC.CMPLSW***
      mask Description: これはAutoCからのブロックです．編集禁止．
  SubSystem1774 : Subsystem  TreatAsAtomicUnit=on
      settings: AttributesFormatString=Attribute946  RTWSystemCode=Function  RTWFcnNameOpts=Use subsystem name
  SubSystem1817 : Subsystem  TreatAsAtomicUnit=on
      settings: AttributesFormatString=Attribute987  RTWSystemCode=Function  RTWFcnNameOpts=Use subsystem name
  wires:
  SubSystem1772/1(# if A) -> SubSystem1817/Trigger
  SubSystem1771/1(# if A) -> SubSystem1774/Trigger

== system lib_all/SubSystem1860 ==
  note: Text368
  Block5095 : Inport
  Block5096 : Inport  Port=2
  Block5097 : Constant  Value=5  OutDataTypeStr=double  SampleTime=-1
  Block5098 : DataStoreRead  DataStoreName=Pair
  Block5099 : Gain  Gain=1/5  SaturateOnIntegerOverflow=on  OutDataTypeStr=Inherit: Same as input  ParamDataTypeStr=Inherit: Same as input
      settings: AttributesFormatString=Attribute1030
  Block5103 : Sum  Inputs=+-  OutDataTypeStr=double  RndMeth=Zero  SaturateOnIntegerOverflow=on  InputSameDT=on
  SubSystem1862 : Subsystem  TreatAsAtomicUnit=on
      settings: RTWSystemCode=Inline
  SubSystem1864 : Subsystem
  Block5128 : Outport
  wires:
  Block5099/1 -> Block5103/2
  Block5103/1 -> Block5128/1
  Block5098/1 -> Block5103/1
  SubSystem1864/1(Block5127) -> Block5099/1
  Block5096/1 -> SubSystem1864/3(Block5106)
  Block5095/1 -> SubSystem1864/2(Block5105)
  Block5097/1 -> SubSystem1862/1(N)
  SubSystem1862/1(action) -> SubSystem1864/Trigger
  SubSystem1862/2(i) -> SubSystem1864/1(Block5104)

== system lib_all/SubSystem1871 ==
  Block5129 : Inport
  Block5130 : Inport  Port=2
  Block5131 : MultiPortSwitch  zeroidx=off  RndMeth=Zero  SaturateOnIntegerOverflow=on  InputSameDT=on
  SubSystem1872 : Subsystem  TreatAsAtomicUnit=on  mask(***ATC.DSRVEC***){bd_version=1.01.A, r0=DSM18, r1=-1, r2=, r3=, r4=, r5=, r6=, r7=, r8=, r9=, c0=, c1=, c2=, c3=, c4=, c5=, c6=, c7=, c8=, c9=}
      mask prompts: bd_version "Version"
      mask help: これはAutoCからのブロックです．編集禁止．
      settings: OpenFcn=warndlg('変更禁止!');
      mask Help: web(ui_blockhelpmng(gcb));
      mask Display: disp('ATC.DSRVEC')
  SubSystem1873 : Subsystem  TreatAsAtomicUnit=on  mask(***ATC.DSRVEC***){bd_version=1.01.A, r0=DSM8, r1=-1, r2=, r3=, r4=, r5=, r6=, r7=, r8=, r9=, c0=, c1=, c2=, c3=, c4=, c5=, c6=, c7=, c8=, c9=}
      mask prompts: bd_version "Version"
      mask help: これはAutoCからのブロックです．編集禁止．
      settings: OpenFcn=warndlg('変更禁止!');
      mask Help: web(ui_blockhelpmng(gcb));
      mask Display: disp('ATC.DSRVEC')
  SubSystem1874 : Subsystem  TreatAsAtomicUnit=on  mask(***ATC.DSRVEC***){bd_version=1.01.A, r0=DSM11, r1=-1, r2=, r3=, r4=, r5=, r6=, r7=, r8=, r9=, c0=, c1=, c2=, c3=, c4=, c5=, c6=, c7=, c8=, c9=}
      mask prompts: bd_version "Version"
      mask help: これはAutoCからのブロックです．編集禁止．
      settings: OpenFcn=warndlg('変更禁止!');
      mask Help: web(ui_blockhelpmng(gcb));
      mask Display: disp('ATC.DSRVEC')
  Block5132 : Outport
  wires:
  Block5129/1 -> Block5131/1
  Block5131/1 -> Block5132/1
  SubSystem1872/1(Output) -> Block5131/2
  SubSystem1873/1(Output) -> Block5131/3
  SubSystem1874/1(Output) -> Block5131/4
  Block5130/1 -> SubSystem1872/1(element), SubSystem1873/1(element), SubSystem1874/1(element)

== system lib_all/SubSystem1875 ==
  Block5133 : Inport
  Block5134 : Inport  Port=2
  Block5135 : MultiPortSwitch  zeroidx=off  RndMeth=Zero  SaturateOnIntegerOverflow=on  InputSameDT=on
  SubSystem1876 : Subsystem  TreatAsAtomicUnit=on  mask(***ATC.DSRVEC***){bd_version=1.01.A, r0=DSM7, r1=-1, r2=, r3=, r4=, r5=, r6=, r7=, r8=, r9=, c0=, c1=, c2=, c3=, c4=, c5=, c6=, c7=, c8=, c9=}
      mask prompts: bd_version "Version"
      mask help: これはAutoCからのブロックです．編集禁止．
      settings: OpenFcn=warndlg('変更禁止!');
      mask Help: web(ui_blockhelpmng(gcb));
      mask Display: disp('ATC.DSRVEC')
  SubSystem1877 : Subsystem  TreatAsAtomicUnit=on  mask(***ATC.DSRVEC***){bd_version=1.01.A, r0=DSM6, r1=-1, r2=, r3=, r4=, r5=, r6=, r7=, r8=, r9=, c0=, c1=, c2=, c3=, c4=, c5=, c6=, c7=, c8=, c9=}
      mask prompts: bd_version "Version"
      mask help: これはAutoCからのブロックです．編集禁止．
      settings: OpenFcn=warndlg('変更禁止!');
      mask Help: web(ui_blockhelpmng(gcb));
      mask Display: disp('ATC.DSRVEC')
  SubSystem1878 : Subsystem  TreatAsAtomicUnit=on  mask(***ATC.DSRVEC***){bd_version=1.01.A, r0=DSM19, r1=-1, r2=, r3=, r4=, r5=, r6=, r7=, r8=, r9=, c0=, c1=, c2=, c3=, c4=, c5=, c6=, c7=, c8=, c9=}
      mask prompts: bd_version "Version"
      mask help: これはAutoCからのブロックです．編集禁止．
      settings: OpenFcn=warndlg('変更禁止!');
      mask Help: web(ui_blockhelpmng(gcb));
      mask Display: disp('ATC.DSRVEC')
  Block5136 : Outport
  wires:
  Block5134/1 -> SubSystem1878/1(element), SubSystem1877/1(element), SubSystem1876/1(element)
  SubSystem1878/1(Output) -> Block5135/4
  SubSystem1877/1(Output) -> Block5135/3
  SubSystem1876/1(Output) -> Block5135/2
  Block5135/1 -> Block5136/1
  Block5133/1 -> Block5135/1

== system lib_all/SubSystem1879 ==
  note: Text376
  note: Text375
  note: Text374
  Block5137 : Inport
  Block5138 : Inport  Port=2
  Block5139 : Inport  Port=3
  Block5140 : Constant  OutDataTypeStr=double  SampleTime=-1
  Block5141 : Gain  Gain=1/5  SaturateOnIntegerOverflow=on  OutDataTypeStr=Inherit: Same as input  ParamDataTypeStr=Inherit: Same as input
      settings: AttributesFormatString=Attribute1040
  Block5142 : Product  OutDataTypeStr=double  SaturateOnIntegerOverflow=on  RndMeth=Zero  InputSameDT=on
  Block5143 : Sum  Inputs=-+  OutDataTypeStr=double  RndMeth=Zero  SaturateOnIntegerOverflow=on  InputSameDT=on
  Block5144 : Sum  OutDataTypeStr=double  RndMeth=Zero  SaturateOnIntegerOverflow=on  InputSameDT=on
  Block5145 : Sum  OutDataTypeStr=double  RndMeth=Zero  SaturateOnIntegerOverflow=on  InputSameDT=on
  SubSystem1881 : Subsystem
  SubSystem1887 : Subsystem
  Block5236 : Outport
  wires:
  Block5145/1 -> SubSystem1887/3(Block5174), SubSystem1881/2(Block5147)
  Block5137/1 -> Block5145/1
  Block5141/1 -> Block5144/2
  Block5142/1 -> Block5141/1
  Block5140/1 -> Block5145/2
  Block5144/1 -> Block5236/1
  SubSystem1881/2(Block5171) -> Block5142/2
  Block5143/1 -> Block5142/1  "SigLabel167"
  SubSystem1887/2(Block5235) -> Block5143/2
  SubSystem1887/1(Block5234) -> Block5143/1, Block5144/1
  Block5139/1 -> SubSystem1887/2(Block5173)
  SubSystem1881/1(Block5170) -> SubSystem1887/1(Block5172)
  Block5138/1 -> SubSystem1881/1(Block5146)

== system lib_all/SubSystem1920 ==
  note: Text384
  note: Text383
  note: Text382
  Block5237 : Inport
  Block5238 : Inport  Port=2
  Block5239 : Inport  Port=3
  Block5240 : Constant  OutDataTypeStr=double  SampleTime=-1
  Block5241 : Gain  Gain=1/5  SaturateOnIntegerOverflow=on  OutDataTypeStr=Inherit: Same as input  ParamDataTypeStr=Inherit: Same as input
      settings: AttributesFormatString=Attribute1056
  Block5242 : Product  OutDataTypeStr=double  SaturateOnIntegerOverflow=on  RndMeth=Zero  InputSameDT=on
  Block5243 : Sum  Inputs=-+  OutDataTypeStr=double  RndMeth=Zero  SaturateOnIntegerOverflow=on  InputSameDT=on
  Block5244 : Sum  OutDataTypeStr=double  RndMeth=Zero  SaturateOnIntegerOverflow=on  InputSameDT=on
  Block5245 : Sum  OutDataTypeStr=double  RndMeth=Zero  SaturateOnIntegerOverflow=on  InputSameDT=on
  SubSystem1922 : Subsystem
  SubSystem1928 : Subsystem
  Block5336 : Outport
  wires:
  Block5238/1 -> SubSystem1922/1(Block5246)
  SubSystem1922/1(Block5270) -> SubSystem1928/1(Block5272)
  Block5239/1 -> SubSystem1928/2(Block5273)
  SubSystem1928/1(Block5334) -> Block5244/1, Block5243/1
  SubSystem1928/2(Block5335) -> Block5243/2
  Block5243/1 -> Block5242/1  "SigLabel170"
  SubSystem1922/2(Block5271) -> Block5242/2
  Block5244/1 -> Block5336/1
  Block5240/1 -> Block5245/2
  Block5242/1 -> Block5241/1
  Block5241/1 -> Block5244/2
  Block5237/1 -> Block5245/1
  Block5245/1 -> SubSystem1922/2(Block5247), SubSystem1928/3(Block5274)

== system lib_all/SubSystem3 ==
  note: Text3
  note: Text2
  Block6 : Inport
  Block7 : Inport  Port=2
  Block8 : MultiPortSwitch  zeroidx=off  RndMeth=Zero  SaturateOnIntegerOverflow=on  InputSameDT=on
  SubSystem4 : Subsystem  TreatAsAtomicUnit=on  mask(***ATC.DSRVEC***){bd_version=1.01.A, r0=DSM10, r1=-1, r2=, r3=, r4=, r5=, r6=, r7=, r8=, r9=, c0=, c1=, c2=, c3=, c4=, c5=, c6=, c7=, c8=, c9=}
      mask prompts: bd_version "Version"
      mask help: これはAutoCからのブロックです．編集禁止．
      settings: OpenFcn=warndlg('変更禁止!');
      mask Help: web(ui_blockhelpmng(gcb));
      mask Display: disp('ATC.DSRVEC')
  SubSystem5 : Subsystem  TreatAsAtomicUnit=on  mask(***ATC.DSRVEC***){bd_version=1.01.A, r0=DSM16, r1=-1, r2=, r3=, r4=, r5=, r6=, r7=, r8=, r9=, c0=, c1=, c2=, c3=, c4=, c5=, c6=, c7=, c8=, c9=}
      mask prompts: bd_version "Version"
      mask help: これはAutoCからのブロックです．編集禁止．
      settings: OpenFcn=warndlg('変更禁止!');
      mask Help: web(ui_blockhelpmng(gcb));
      mask Display: disp('ATC.DSRVEC')
  SubSystem6 : Subsystem  TreatAsAtomicUnit=on  mask(***ATC.DSRVEC***){bd_version=1.01.A, r0=DSM17, r1=-1, r2=, r3=, r4=, r5=, r6=, r7=, r8=, r9=, c0=, c1=, c2=, c3=, c4=, c5=, c6=, c7=, c8=, c9=}
      mask prompts: bd_version "Version"
      mask help: これはAutoCからのブロックです．編集禁止．
      settings: OpenFcn=warndlg('変更禁止!');
      mask Help: web(ui_blockhelpmng(gcb));
      mask Display: disp('ATC.DSRVEC')
  Block9 : Outport
  wires:
  Block8/1 -> Block9/1
  Block7/1 -> SubSystem6/1(element), SubSystem5/1(element), SubSystem4/1(element)
  Block6/1 -> Block8/1
  SubSystem4/1(Output) -> Block8/2
  SubSystem5/1(Output) -> Block8/3
  SubSystem6/1(Output) -> Block8/4

== system lib_all/SubSystem347 ==
  note: Text74
  note: Text73
  note: Text72
  Block761 : Inport
  SubSystem349 : Subsystem  Priority=1  TreatAsAtomicUnit=on
      settings: AttributesFormatString=Attribute165  RTWSystemCode=Inline
  SubSystem477 : Subsystem  Priority=2  TreatAsAtomicUnit=on
      settings: AttributesFormatString=Attribute218  RTWSystemCode=Inline
  SubSystem503 : Subsystem  Priority=3  TreatAsAtomicUnit=on
      settings: AttributesFormatString=Attribute235  RTWSystemCode=Inline
  wires:
  Block761/1 -> SubSystem477/1(Block1065), SubSystem349/1(Block762)

== system lib_all/SubSystem518 ==
  note: Text90
  Block1136 : Inport
  SubSystem520 : Subsystem  TreatAsAtomicUnit=on
      settings: RTWSystemCode=Inline
  SubSystem523 : Subsystem  TreatAsAtomicUnit=on
      settings: RTWSystemCode=Inline
  SubSystem630 : Subsystem  TreatAsAtomicUnit=on
      settings: RTWSystemCode=Inline
  wires:
  Block1136/1 -> SubSystem630/1(Block1409), SubSystem523/1(Block1156), SubSystem520/1(Block1137)
  SubSystem523/2(Block1408) -> SubSystem630/3(Block1411)
  SubSystem523/1(Block1407) -> SubSystem630/2(Block1410)
  SubSystem520/2(Block1155) -> SubSystem523/3(Block1158)
  SubSystem520/1(Block1154) -> SubSystem523/2(Block1157)

== system lib_all/SubSystem643 ==
  SubSystem645 : Subsystem  TreatAsAtomicUnit=on  RTWFileName=filename
      settings: RTWSystemCode=Function  RTWFcnNameOpts=Use subsystem name

== system lib_all/SubSystem657 ==
  note: Text92
  note: Text91
  Block1448 : Inport
  Block1449 : Inport  Port=2
  Block1450 : Inport  Port=3
  Block1451 : Constant  OutDataTypeStr=double  SampleTime=-1
  Block1452 : Sum  Inputs=+-|  OutDataTypeStr=double  RndMeth=Zero  SaturateOnIntegerOverflow=on  InputSameDT=on
  Block1453 : Sum  Inputs=|++  OutDataTypeStr=double  RndMeth=Zero  SaturateOnIntegerOverflow=on  InputSameDT=on
  SubSystem658 : Subsystem  TreatAsAtomicUnit=on  mask(***ATC.DIVISION***){bd_version=1.01.A, r0=チェック無し, r1=, r2=, r3=, r4=, r5=, r6=, r7=, r8=, r9=, c0=, c1=, c2=, c3=, c4=, c5=, c6=, c7=, c8=, c9=}
      mask prompts: bd_version "Version"
      mask help: これはAutoCからのブロックです．編集禁止．
      settings: OpenFcn=warndlg('変更禁止!');
      mask Help: web(ui_blockhelpmng(gcb));
  SubSystem659 : Subsystem  TreatAsAtomicUnit=on  mask(***ATC.SATURATION***){bd_version=1.01.A, r0=下限ガード, r1=下限ガード, r2=, r3=, r4=, r5=, r6=, r7=, r8=, r9=, c0=, c1=, c2=, c3=, c4=, c5=, c6=, c7=, c8=, c9=}
      mask prompts: bd_version "Version"
      mask help: これはAutoCからのブロックです．編集禁止．
      settings: OpenFcn=warndlg('変更禁止!');  RTWSystemCode=Inline
      mask Help: web(ui_blockhelpmng(gcb));
      mask Display:
      | plot([0 1],[0.65 0.65])
      | plot([0.5 0.5],[0.2 1])
      | plot([0.2 0.8],[0.3 1])
      | plot([0 0.2],[0.3 0.3])
      | plot([0 0],[0 0])
      | text(0.06,0.15,sprintf('Lower'))
  Block1454 : Outport
  wires:
  Block1448/1 -> Block1452/1
  Block1450/1 -> SubSystem659/1(Input)
  SubSystem659/1(Output) -> SubSystem658/2(div)
  Block1452/1 -> SubSystem658/1(mult)
  SubSystem658/1(Out) -> Block1453/2
  Block1453/1 -> Block1454/1
  Block1449/1 -> Block1452/2, Block1453/1
  Block1451/1 -> SubSystem659/2(Min)

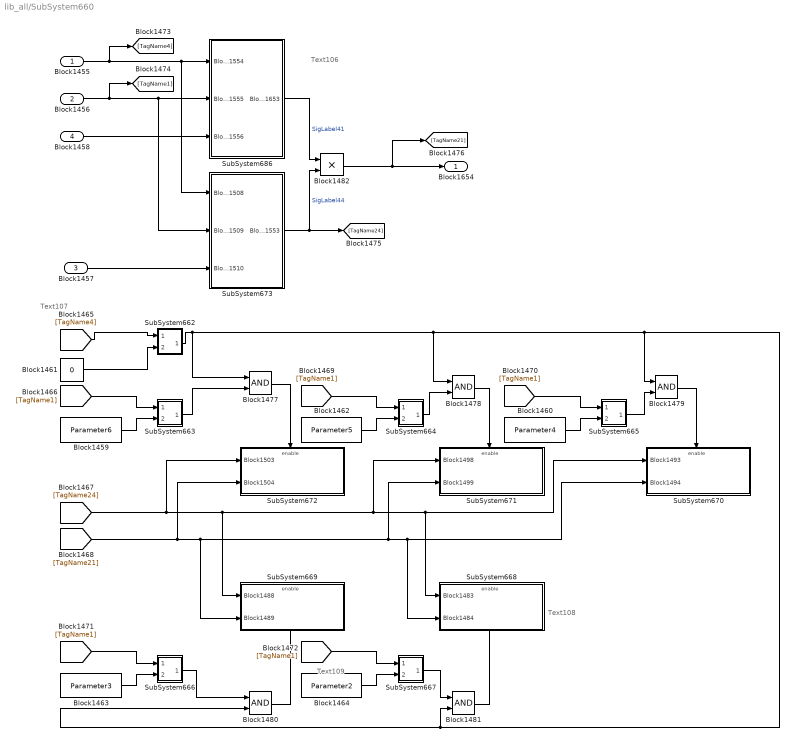
== system lib_all/SubSystem660 ==
  note: Text109
  note: Text108
  note: Text107
  note: Text106
  Block1455 : Inport
  Block1456 : Inport  Port=2
  Block1457 : Inport  Port=3
  Block1458 : Inport  Port=4
  Block1459 : Constant  Value=Parameter6  OutDataTypeStr=double  SampleTime=-1
  Block1460 : Constant  Value=Parameter4  OutDataTypeStr=double  SampleTime=-1
  Block1461 : Constant  Value=0  OutDataTypeStr=double  SampleTime=-1
  Block1462 : Constant  Value=Parameter5  OutDataTypeStr=double  SampleTime=-1
  Block1463 : Constant  Value=Parameter3  OutDataTypeStr=double  SampleTime=-1
  Block1464 : Constant  Value=Parameter2  OutDataTypeStr=double  SampleTime=-1
  Block{1477..1481} (5 alike) : Logic
  Block1482 : Product  OutDataTypeStr=double  SaturateOnIntegerOverflow=on  RndMeth=Zero  InputSameDT=on
  SubSystem662 : Subsystem
  SubSystem663 : Subsystem
  SubSystem664 : Subsystem
  SubSystem665 : Subsystem
  SubSystem666 : Subsystem
  SubSystem667 : Subsystem
  SubSystem668 : Subsystem
      settings: PropExecContextOutsideSubsystem=on
  SubSystem669 : Subsystem
      settings: PropExecContextOutsideSubsystem=on
  SubSystem670 : Subsystem
      settings: PropExecContextOutsideSubsystem=on
  SubSystem671 : Subsystem
      settings: PropExecContextOutsideSubsystem=on
  SubSystem672 : Subsystem
      settings: PropExecContextOutsideSubsystem=on
  SubSystem673 : Subsystem  Priority=2  TreatAsAtomicUnit=on
      settings: AttributesFormatString=Attribute313  RTWSystemCode=Function  RTWFcnNameOpts=Use subsystem name
  SubSystem686 : Subsystem  Priority=1  TreatAsAtomicUnit=on
      settings: AttributesFormatString=Attribute326  RTWFcnNameOpts=Use subsystem name
  Block1654 : Outport
  wires:
  Block1479/1 -> SubSystem670/Enable
  SubSystem667/1(Out1) -> Block1481/1
  SubSystem666/1(Out1) -> Block1480/1
  Block1481/1 -> SubSystem668/Enable
  Block1480/1 -> SubSystem669/Enable
  Block1464/1 -> SubSystem667/2(In2)
  [TagName1] -> SubSystem667/1(In1)
  Block1463/1 -> SubSystem666/2(In2)
  [TagName1] -> SubSystem666/1(In1)
  [TagName21] -> SubSystem670/2(Block1494), SubSystem668/2(Block1484), SubSystem671/2(Block1499), SubSystem669/2(Block1489), SubSystem672/2(Block1504)
  [TagName24] -> SubSystem669/1(Block1488), SubSystem671/1(Block1498), SubSystem670/1(Block1493), SubSystem668/1(Block1483), SubSystem672/1(Block1503)
  Block1478/1 -> SubSystem671/Enable
  SubSystem665/1(Out1) -> Block1479/2
  Block1460/1 -> SubSystem665/2(In2)
  [TagName1] -> SubSystem665/1(In1)
  Block1477/1 -> SubSystem672/Enable
  SubSystem664/1(Out1) -> Block1478/2
  Block1462/1 -> SubSystem664/2(In2)
  [TagName1] -> SubSystem664/1(In1)
  SubSystem663/1(Out1) -> Block1477/2
  SubSystem662/1(Out1) -> Block1480/2, Block1481/2, Block1479/1, Block1478/1, Block1477/1
  Block1459/1 -> SubSystem663/2(In2)
  [TagName1] -> SubSystem663/1(In1)
  Block1461/1 -> SubSystem662/2(In2)
  [TagName4] -> SubSystem662/1(In1)
  Block1482/1 -> [TagName21], Block1654/1
  SubSystem673/1(Block1553) -> [TagName24], Block1482/2  "SigLabel44"
  SubSystem686/1(Block1653) -> Block1482/1  "SigLabel41"
  Block1458/1 -> SubSystem686/3(Block1556)
  Block1455/1 -> [TagName4], SubSystem673/1(Block1508), SubSystem686/1(Block1554)
  Block1456/1 -> [TagName1], SubSystem673/2(Block1509), SubSystem686/2(Block1555)
  Block1457/1 -> SubSystem673/3(Block1510)

== system lib_all/SubSystem7 ==
  note: Text29
  note: Text28
  note: Text27
  Block10 : Inport
  SubSystem134 : Subsystem  Priority=2  TreatAsAtomicUnit=on
      settings: AttributesFormatString=Attribute54  RTWSystemCode=Inline
  SubSystem161 : Subsystem  Priority=3  TreatAsAtomicUnit=on
      settings: AttributesFormatString=Attribute72  RTWSystemCode=Inline
  SubSystem9 : Subsystem  Priority=1  TreatAsAtomicUnit=on
      settings: AttributesFormatString=Attribute3  RTWSystemCode=Inline
  wires:
  Block10/1 -> SubSystem9/1(Block11), SubSystem134/1(Block311)
